simulink model "HvacMdlAirTDistbnDynFildFrnt"  (R2022b)
settings: Solver=FixedStepDiscrete  StartTime=0.0  StopTime=10.0  FixedStep=0.2  RelTol=1e-3
(part 1 of 2)
settings Model: Creator=blue.ge  LastModifiedBy=blue.ge
settings DataIOCC: SaveFormat=StructureWithTime
settings OptimizationCC: DefaultParameterBehavior=Inlined  EnhancedBackFolding=on  PassReuseOutputArgsAs=Individual arguments  ZeroExternalMemoryAtStartup=off  ZeroInternalMemoryAtStartup=off  OptimizeBlockOrder=speed  DifferentSizesBufferReuse=on  OptimizationCustomize=off  EfficientTunableParamExpr=on
settings DebuggingCC: ParameterTunabilityLossMsg=error
settings ModelReferenceCC: ModelReferenceNumInstancesAllowed=Single
settings SFSimCC: MATLABDynamicMemAlloc=off
settings RTWCC: Description=AUTOSAR  SystemTargetFile=autosar.tlc  GenCodeOnly=on  TemplateMakefile=ert_default_tmf
settings CodeAppCC: ForceParamTrailComments=on  IgnoreCustomStorageClasses=off  OperatorAnnotations=on  SimulinkDataObjDesc=on  SFDataObjDesc=on  InsertBlockDesc=on
settings STFCustomTargetCC: TargetLangStandard=C99 (ISO)  MultiwordLength=256  ParMdlRefBuildCompliant=on  CompOptLevelCompliant=on  IncludeMdlTerminateFcn=off  CombineOutputUpdateFcns=on  SuppressErrorStatus=on  ERTCustomFileBanners=on  SupportNonFinite=off  SupportComplex=off  SupportContinuousTime=off  SupportNonInlinedSFcns=off  RemoveResetFunc=on  MATLABClassNameForMDSCustomization=Simulink.SoftwareTarget.ERTCustomization  AutosarCompliant=on  UseToolchainInfoCompliant=on  LookupTableObjectStructAxisOrder=2,1,3,4,...  LUTObjectStructOrderEvenSpacing=Size,Table,Breakpoints  SystemTargetFile=autosar.tlc
settings ERTTargetCC: TargetLangStandard=C99 (ISO)  ConcurrentExecutionCompliant=off  IncludeMdlTerminateFcn=off  SuppressErrorStatus=on  SupportNonFinite=off  SupportComplex=off  ModelStepFunctionPrototypeControlCompliant=off  CPPClassGenCompliant=off  AutosarCompliant=on  LookupTableObjectStructAxisOrder=2,1,3,4,...  LUTObjectStructOrderEvenSpacing=Size,Table,Breakpoints  InlinedParameterPlacement=NonHierarchical  ERTCustomFileTemplate=example_file_process.tlc

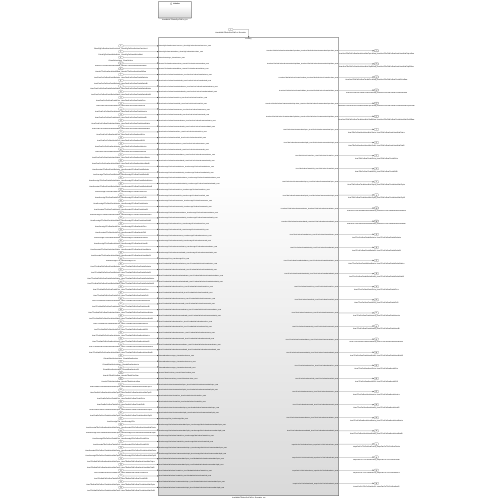
[thumbnail: Simulink's stored preview of the top-level diagram]
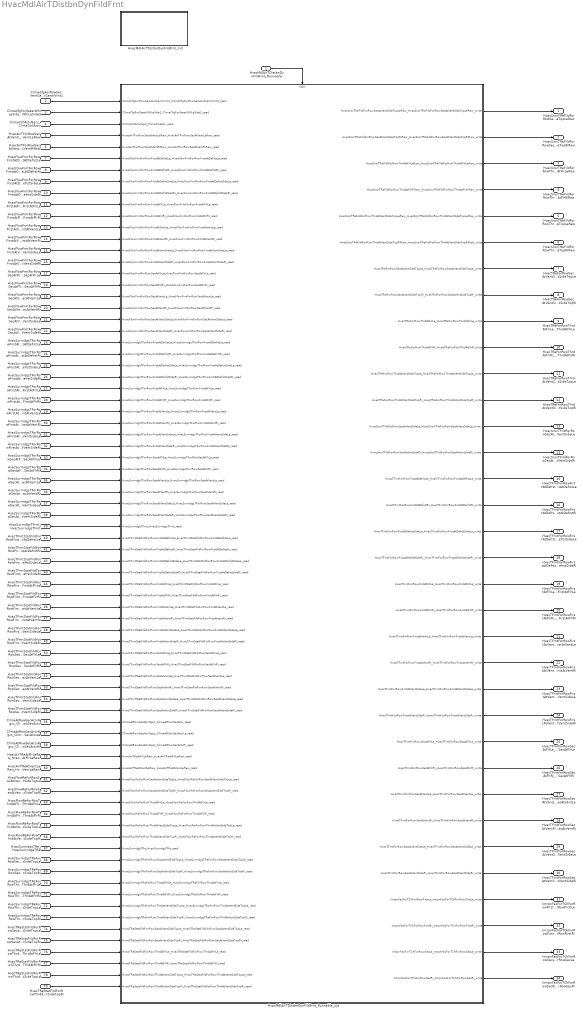
== system HvacMdlAirTDistbnDynFildFrnt ==
  HvacMdlAirTDistbnDynFildFrnt_Runnable : Inport  SampleTime=0.2  OutputFunctionCall=on
  ClimaCfgForRowSecVentCentrVrnt1_ClimaCfgForRowSecVentCentrVrnt1 : Inport  Port=2  OutDataTypeStr=Enum: ClimaCfgForRowSecVentCentrVrnt1  PortDimensions=1
  ClimaCfgForSteerWhlLeSide1_ClimaCfgForSteerWhlLeSide1 : Inport  Port=3  OutDataTypeStr=Boolean  PortDimensions=1
  ClimaCtrlActvNgcc_ClimaCtrlActv : Inport  Port=4  OutDataTypeStr=Boolean  PortDimensions=1
  HvacAirTForRowSecAtVentLeRaw_HvacAirTForRowSecAtVentLeRaw : Inport  Port=5  OutDataTypeStr=Bus: HvacAirTForRowSecAtVentLe  PortDimensions=1  BusOutputAsStruct=on
  HvacAirTForRowSecAtVentRiRaw_HvacAirTForRowSecAtVentRiRaw : Inport  Port=6  OutDataTypeStr=Bus: HvacAirTForRowSecAtVentRi  PortDimensions=1  BusOutputAsStruct=on
  HvacFlowFrntForRowFirstAtDefrstLe_HvacFlowFrntForRowFirstAtDefrstLe : Inport  Port=7  OutDataTypeStr=AirFlow30  PortDimensions=1
  HvacFlowFrntForRowFirstAtDefrstRi_HvacFlowFrntForRowFirstAtDefrstRi : Inport  Port=8  OutDataTypeStr=AirFlow30  PortDimensions=1
  HvacFlowFrntForRowFirstAtDefrstSideLe_HvacFlowFrntForRowFirstAtDefrstSideLe : Inport  Port=9  OutDataTypeStr=AirFlow30  PortDimensions=1
  HvacFlowFrntForRowFirstAtDefrstSideRi_HvacFlowFrntForRowFirstAtDefrstSideRi : Inport  Port=10  OutDataTypeStr=AirFlow30  PortDimensions=1
  HvacFlowFrntForRowFirstAtFlrLe_HvacFlowFrntForRowFirstAtFlrLe : Inport  Port=11  OutDataTypeStr=AirFlow30  PortDimensions=1
  HvacFlowFrntForRowFirstAtFlrRi_HvacFlowFrntForRowFirstAtFlrRi : Inport  Port=12  OutDataTypeStr=AirFlow30  PortDimensions=1
  HvacFlowFrntForRowFirstAtVentLe_HvacFlowFrntForRowFirstAtVentLe : Inport  Port=13  OutDataTypeStr=AirFlow30  PortDimensions=1
  HvacFlowFrntForRowFirstAtVentRi_HvacFlowFrntForRowFirstAtVentRi : Inport  Port=14  OutDataTypeStr=AirFlow30  PortDimensions=1
  HvacFlowFrntForRowFirstAtVentSideLe_HvacFlowFrntForRowFirstAtVentSideLe : Inport  Port=15  OutDataTypeStr=AirFlow30  PortDimensions=1
  HvacFlowFrntForRowFirstAtVentSideRi_HvacFlowFrntForRowFirstAtVentSideRi : Inport  Port=16  OutDataTypeStr=AirFlow30  PortDimensions=1
  HvacFlowFrntForRowSecAtFlrLe_HvacFlowFrntForRowSecAtFlrLe : Inport  Port=17  OutDataTypeStr=AirFlow30  PortDimensions=1
  HvacFlowFrntForRowSecAtFlrRi_HvacFlowFrntForRowSecAtFlrRi : Inport  Port=18  OutDataTypeStr=AirFlow30  PortDimensions=1
  HvacFlowFrntForRowSecAtVentLe_HvacFlowFrntForRowSecAtVentLe : Inport  Port=19  OutDataTypeStr=AirFlow30  PortDimensions=1
  HvacFlowFrntForRowSecAtVentRi_HvacFlowFrntForRowSecAtVentRi : Inport  Port=20  OutDataTypeStr=AirFlow30  PortDimensions=1
  HvacFlowFrntForRowSecAtVentSideLe_HvacFlowFrntForRowSecAtVentSideLe : Inport  Port=21  OutDataTypeStr=AirFlow30  PortDimensions=1
  HvacFlowFrntForRowSecAtVentSideRi_HvacFlowFrntForRowSecAtVentSideRi : Inport  Port=22  OutDataTypeStr=AirFlow30  PortDimensions=1
  HvacSurrndgsTForRowFirstAtDefrstLe_HvacSurrndgsTForRowFirstAtDefrstLe : Inport  Port=23  OutDataTypeStr=T30  PortDimensions=1
  HvacSurrndgsTForRowFirstAtDefrstRi_HvacSurrndgsTForRowFirstAtDefrstRi : Inport  Port=24  OutDataTypeStr=T30  PortDimensions=1
  HvacSurrndgsTForRowFirstAtDefrstSideLe_HvacSurrndgsTForRowFirstAtDefrstSideLe : Inport  Port=25  OutDataTypeStr=T30  PortDimensions=1
  HvacSurrndgsTForRowFirstAtDefrstSideRi_HvacSurrndgsTForRowFirstAtDefrstSideRi : Inport  Port=26  OutDataTypeStr=T30  PortDimensions=1
  HvacSurrndgsTForRowFirstAtFlrLe_HvacSurrndgsTForRowFirstAtFlrLe : Inport  Port=27  OutDataTypeStr=T30  PortDimensions=1
  HvacSurrndgsTForRowFirstAtFlrRi_HvacSurrndgsTForRowFirstAtFlrRi : Inport  Port=28  OutDataTypeStr=T30  PortDimensions=1
  HvacSurrndgsTForRowFirstAtVentLe_HvacSurrndgsTForRowFirstAtVentLe : Inport  Port=29  OutDataTypeStr=T30  PortDimensions=1
  HvacSurrndgsTForRowFirstAtVentRi_HvacSurrndgsTForRowFirstAtVentRi : Inport  Port=30  OutDataTypeStr=T30  PortDimensions=1
  HvacSurrndgsTForRowFirstAtVentSideLe_HvacSurrndgsTForRowFirstAtVentSideLe : Inport  Port=31  OutDataTypeStr=T30  PortDimensions=1
  HvacSurrndgsTForRowFirstAtVentSideRi_HvacSurrndgsTForRowFirstAtVentSideRi : Inport  Port=32  OutDataTypeStr=T30  PortDimensions=1
  HvacSurrndgsTForRowSecAtFlrLe_HvacSurrndgsTForRowSecAtFlrLe : Inport  Port=33  OutDataTypeStr=T30  PortDimensions=1
  HvacSurrndgsTForRowSecAtFlrRi_HvacSurrndgsTForRowSecAtFlrRi : Inport  Port=34  OutDataTypeStr=T30  PortDimensions=1
  HvacSurrndgsTForRowSecAtVentLe_HvacSurrndgsTForRowSecAtVentLe : Inport  Port=35  OutDataTypeStr=T30  PortDimensions=1
  HvacSurrndgsTForRowSecAtVentRi_HvacSurrndgsTForRowSecAtVentRi : Inport  Port=36  OutDataTypeStr=T30  PortDimensions=1
  HvacSurrndgsTForRowSecAtVentSideLe_HvacSurrndgsTForRowSecAtVentSideLe : Inport  Port=37  OutDataTypeStr=T30  PortDimensions=1
  HvacSurrndgsTForRowSecAtVentSideRi_HvacSurrndgsTForRowSecAtVentSideRi : Inport  Port=38  OutDataTypeStr=T30  PortDimensions=1
  HvacSurrndgsTFrnt_HvacSurrndgsTFrnt : Inport  Port=39  OutDataTypeStr=T30  PortDimensions=1
  HvacTFrntStatFildForRowFirstAtDefrstLe_HvacTFrntStatFildForRowFirstAtDefrstLe : Inport  Port=40  OutDataTypeStr=T30  PortDimensions=1
  HvacTFrntStatFildForRowFirstAtDefrstRi_HvacTFrntStatFildForRowFirstAtDefrstRi : Inport  Port=41  OutDataTypeStr=T30  PortDimensions=1
  HvacTFrntStatFildForRowFirstAtDefrstSideLe_HvacTFrntStatFildForRowFirstAtDefrstSideLe : Inport  Port=42  OutDataTypeStr=T30  PortDimensions=1
  HvacTFrntStatFildForRowFirstAtDefrstSideRi_HvacTFrntStatFildForRowFirstAtDefrstSideRi : Inport  Port=43  OutDataTypeStr=T30  PortDimensions=1
  HvacTFrntStatFildForRowFirstAtFlrLe_HvacTFrntStatFildForRowFirstAtFlrLe : Inport  Port=44  OutDataTypeStr=T30  PortDimensions=1
  HvacTFrntStatFildForRowFirstAtFlrRi_HvacTFrntStatFildForRowFirstAtFlrRi : Inport  Port=45  OutDataTypeStr=T30  PortDimensions=1
  HvacTFrntStatFildForRowFirstAtVentLe_HvacTFrntStatFildForRowFirstAtVentLe : Inport  Port=46  OutDataTypeStr=T30  PortDimensions=1
  HvacTFrntStatFildForRowFirstAtVentRi_HvacTFrntStatFildForRowFirstAtVentRi : Inport  Port=47  OutDataTypeStr=T30  PortDimensions=1
  HvacTFrntStatFildForRowFirstAtVentSideLe_HvacTFrntStatFildForRowFirstAtVentSideLe : Inport  Port=48  OutDataTypeStr=T30  PortDimensions=1
  HvacTFrntStatFildForRowFirstAtVentSideRi_HvacTFrntStatFildForRowFirstAtVentSideRi : Inport  Port=49  OutDataTypeStr=T30  PortDimensions=1
  HvacTFrntStatFildForRowSecAtFlrLe_HvacTFrntStatFildForRowSecAtFlrLe : Inport  Port=50  OutDataTypeStr=T30  PortDimensions=1
  HvacTFrntStatFildForRowSecAtFlrRi_HvacTFrntStatFildForRowSecAtFlrRi : Inport  Port=51  OutDataTypeStr=T30  PortDimensions=1
  HvacTFrntStatFildForRowSecAtVentLe_HvacTFrntStatFildForRowSecAtVentLe : Inport  Port=52  OutDataTypeStr=T30  PortDimensions=1
  HvacTFrntStatFildForRowSecAtVentRi_HvacTFrntStatFildForRowSecAtVentRi : Inport  Port=53  OutDataTypeStr=T30  PortDimensions=1
  HvacTFrntStatFildForRowSecAtVentSideLe_HvacTFrntStatFildForRowSecAtVentSideLe : Inport  Port=54  OutDataTypeStr=T30  PortDimensions=1
  HvacTFrntStatFildForRowSecAtVentSideRi_HvacTFrntStatFildForRowSecAtVentSideRi : Inport  Port=55  OutDataTypeStr=T30  PortDimensions=1
  ClimaAtRowSecActvNgcc_ClimaAtRowSecActv : Inport  Port=56  OutDataTypeStr=Boolean  PortDimensions=1
  ClimaAtRowSecActvNgcc_ClimaAtRowSecActvLe : Inport  Port=57  OutDataTypeStr=Boolean  PortDimensions=1
  ClimaAtRowSecActvNgcc_ClimaAtRowSecActvRi : Inport  Port=58  OutDataTypeStr=Boolean  PortDimensions=1
  HvacAirTReAtFlrLeRaw_HvacAirTReAtFlrLeRaw : Inport  Port=59  OutDataTypeStr=Bus: HvacAirTReAtFlrLe  PortDimensions=1  BusOutputAsStruct=on
  HvacAirTReAtVentLeRaw_HvacAirTReAtVentLeRaw : Inport  Port=60  OutDataTypeStr=Bus: HvacAirTReAtVentLe  PortDimensions=1  BusOutputAsStruct=on
  HvacFlowReForRowSecAtVentSideTopLe_HvacFlowReForRowSecAtVentSideTopLe : Inport  Port=61  OutDataTypeStr=AirFlow30  PortDimensions=1
  HvacFlowReForRowSecAtVentSideTopRi_HvacFlowReForRowSecAtVentSideTopRi : Inport  Port=62  OutDataTypeStr=AirFlow30  PortDimensions=1
  HvacFlowReForRowThrdAtFlrLe_HvacFlowReForRowThrdAtFlrLe : Inport  Port=63  OutDataTypeStr=AirFlow30  PortDimensions=1
  HvacFlowReForRowThrdAtFlrRi_HvacFlowReForRowThrdAtFlrRi : Inport  Port=64  OutDataTypeStr=AirFlow30  PortDimensions=1
  HvacFlowReForRowThrdAtVentSideTopLe_HvacFlowReForRowThrdAtVentSideTopLe : Inport  Port=65  OutDataTypeStr=AirFlow30  PortDimensions=1
  HvacFlowReForRowThrdAtVentSideTopRi_HvacFlowReForRowThrdAtVentSideTopRi : Inport  Port=66  OutDataTypeStr=AirFlow30  PortDimensions=1
  HvacSurrndgsTRe_HvacSurrndgsTRe : Inport  Port=67  OutDataTypeStr=T30  PortDimensions=1
  HvacSurrndgsTReForRowSecAtVentSideTopLe_HvacSurrndgsTReForRowSecAtVentSideTopLe : Inport  Port=68  OutDataTypeStr=T30  PortDimensions=1
  HvacSurrndgsTReForRowSecAtVentSideTopRi_HvacSurrndgsTReForRowSecAtVentSideTopRi : Inport  Port=69  OutDataTypeStr=T30  PortDimensions=1
  HvacSurrndgsTReForRowThrdAtFlrLe_HvacSurrndgsTReForRowThrdAtFlrLe : Inport  Port=70  OutDataTypeStr=T30  PortDimensions=1
  HvacSurrndgsTReForRowThrdAtFlrRi_HvacSurrndgsTReForRowThrdAtFlrRi : Inport  Port=71  OutDataTypeStr=T30  PortDimensions=1
  HvacSurrndgsTReForRowThrdAtVentSideTopLe_HvacSurrndgsTReForRowThrdAtVentSideTopLe : Inport  Port=72  OutDataTypeStr=T30  PortDimensions=1
  HvacSurrndgsTReForRowThrdAtVentSideTopRi_HvacSurrndgsTReForRowThrdAtVentSideTopRi : Inport  Port=73  OutDataTypeStr=T30  PortDimensions=1
  HvacTReStatFildForRowSecAtVentSideTopLe_HvacTReStatFildForRowSecAtVentSideTopLe : Inport  Port=74  OutDataTypeStr=T30  PortDimensions=1
  HvacTReStatFildForRowSecAtVentSideTopRi_HvacTReStatFildForRowSecAtVentSideTopRi : Inport  Port=75  OutDataTypeStr=T30  PortDimensions=1
  HvacTReStatFildForRowThrdAtFlrLe_HvacTReStatFildForRowThrdAtFlrLe : Inport  Port=76  OutDataTypeStr=T30  PortDimensions=1
  HvacTReStatFildForRowThrdAtFlrRi_HvacTReStatFildForRowThrdAtFlrRi : Inport  Port=77  OutDataTypeStr=T30  PortDimensions=1
  HvacTReStatFildForRowThrdAtVentSideTopLe_HvacTReStatFildForRowThrdAtVentSideTopLe : Inport  Port=78  OutDataTypeStr=T30  PortDimensions=1
  HvacTReStatFildForRowThrdAtVentSideTopRi_HvacTReStatFildForRowThrdAtVentSideTopRi : Inport  Port=79  OutDataTypeStr=T30  PortDimensions=1
  HvacMdlAirTDistbnDynFildFrnt_Init : Subsystem
  HvacMdlAirTDistbnDynFildFrnt_Runnable_sys : Subsystem
  HvacSnsrTReFildForRowSecAtVentSideTopLeRaw_HvacSnsrTReFildForRowSecAtVentSideTopLeRaw : Outport  OutDataTypeStr=Bus: HvacAirTReForRowSecAtVentSideTopLe  PortDimensions=1  BusOutputAsStruct=on
  HvacSnsrTReFildForRowSecAtVentSideTopRiRaw_HvacSnsrTReFildForRowSecAtVentSideTopRiRaw : Outport  Port=2  OutDataTypeStr=Bus: HvacAirTReForRowSecAtVentSideTopRi  PortDimensions=1  BusOutputAsStruct=on
  HvacSnsrTReFildForRowThrdAtFlrLeRaw_HvacSnsrTReFildForRowThrdAtFlrLeRaw : Outport  Port=3  OutDataTypeStr=Bus: HvacAirTReForRowThrdAtFlrLe  PortDimensions=1  BusOutputAsStruct=on
  HvacSnsrTReFildForRowThrdAtFlrRiRaw_HvacSnsrTReFildForRowThrdAtFlrRiRaw : Outport  Port=4  OutDataTypeStr=Bus: HvacAirTReForRowThrdAtFlrRi  PortDimensions=1  BusOutputAsStruct=on
  HvacSnsrTReFildForRowThrdAtVentSideTopLeRaw_HvacSnsrTReFildForRowThrdAtVentSideTopLeRaw : Outport  Port=5  OutDataTypeStr=Bus: HvacAirTReForRowThrdAtVentSideTopLe  PortDimensions=1  BusOutputAsStruct=on
  HvacSnsrTReFildForRowThrdAtVentSideTopRiRaw_HvacSnsrTReFildForRowThrdAtVentSideTopRiRaw : Outport  Port=6  OutDataTypeStr=Bus: HvacAirTReForRowThrdAtVentSideTopRi  PortDimensions=1  BusOutputAsStruct=on
  HvacTReForRowSecAtVentSideTopLe_HvacTReForRowSecAtVentSideTopLe : Outport  Port=7  OutDataTypeStr=T30  PortDimensions=1
  HvacTReForRowSecAtVentSideTopRi_HvacTReForRowSecAtVentSideTopRi : Outport  Port=8  OutDataTypeStr=T30  PortDimensions=1
  HvacTReForRowThrdAtFlrLe_HvacTReForRowThrdAtFlrLe : Outport  Port=9  OutDataTypeStr=T30  PortDimensions=1
  HvacTReForRowThrdAtFlrRi_HvacTReForRowThrdAtFlrRi : Outport  Port=10  OutDataTypeStr=T30  PortDimensions=1
  HvacTReForRowThrdAtVentSideTopLe_HvacTReForRowThrdAtVentSideTopLe : Outport  Port=11  OutDataTypeStr=T30  PortDimensions=1
  HvacTReForRowThrdAtVentSideTopRi_HvacTReForRowThrdAtVentSideTopRi : Outport  Port=12  OutDataTypeStr=T30  PortDimensions=1
  HvacSnsrTFildForRowSecAtVentSideLe_HvacSnsrTFildForRowSecAtVentSideLe : Outport  Port=13  OutDataTypeStr=Bus: HvacAirTForRowSecAtVentLe  PortDimensions=1  BusOutputAsStruct=on
  HvacSnsrTFildForRowSecAtVentSideRi_HvacSnsrTFildForRowSecAtVentSideRi : Outport  Port=14  OutDataTypeStr=Bus: HvacAirTForRowSecAtVentRi  PortDimensions=1  BusOutputAsStruct=on
  HvacTFrntForRowFirstAtDefrstLe_HvacTFrntForRowFirstAtDefrstLe : Outport  Port=15  OutDataTypeStr=T30  PortDimensions=1
  HvacTFrntForRowFirstAtDefrstRi_HvacTFrntForRowFirstAtDefrstRi : Outport  Port=16  OutDataTypeStr=T30  PortDimensions=1
  HvacTFrntForRowFirstAtDefrstSideLe_HvacTFrntForRowFirstAtDefrstSideLe : Outport  Port=17  OutDataTypeStr=T30  PortDimensions=1
  HvacTFrntForRowFirstAtDefrstSideRi_HvacTFrntForRowFirstAtDefrstSideRi : Outport  Port=18  OutDataTypeStr=T30  PortDimensions=1
  HvacTFrntForRowFirstAtFlrLe_HvacTFrntForRowFirstAtFlrLe : Outport  Port=19  OutDataTypeStr=T30  PortDimensions=1
  HvacTFrntForRowFirstAtFlrRi_HvacTFrntForRowFirstAtFlrRi : Outport  Port=20  OutDataTypeStr=T30  PortDimensions=1
  HvacTFrntForRowFirstAtVentLe_HvacTFrntForRowFirstAtVentLe : Outport  Port=21  OutDataTypeStr=T30  PortDimensions=1
  HvacTFrntForRowFirstAtVentRi_HvacTFrntForRowFirstAtVentRi : Outport  Port=22  OutDataTypeStr=T30  PortDimensions=1
  HvacTFrntForRowFirstAtVentSideLe_HvacTFrntForRowFirstAtVentSideLe : Outport  Port=23  OutDataTypeStr=T30  PortDimensions=1
  HvacTFrntForRowFirstAtVentSideRi_HvacTFrntForRowFirstAtVentSideRi : Outport  Port=24  OutDataTypeStr=T30  PortDimensions=1
  HvacTFrntForRowSecAtFlrLe_HvacTFrntForRowSecAtFlrLe : Outport  Port=25  OutDataTypeStr=T30  PortDimensions=1
  HvacTFrntForRowSecAtFlrRi_HvacTFrntForRowSecAtFlrRi : Outport  Port=26  OutDataTypeStr=T30  PortDimensions=1
  HvacTFrntForRowSecAtVentLe_HvacTFrntForRowSecAtVentLe : Outport  Port=27  OutDataTypeStr=T30  PortDimensions=1
  HvacTFrntForRowSecAtVentRi_HvacTFrntForRowSecAtVentRi : Outport  Port=28  OutDataTypeStr=T30  PortDimensions=1
  HvacTFrntForRowSecAtVentSideLe_HvacTFrntForRowSecAtVentSideLe : Outport  Port=29  OutDataTypeStr=T30  PortDimensions=1
  HvacTFrntForRowSecAtVentSideRi_HvacTFrntForRowSecAtVentSideRi : Outport  Port=30  OutDataTypeStr=T30  PortDimensions=1
  IntrpnFacForTChForRowFirstLe_IntrpnFacForTChForRowFirstLe : Outport  Port=31  OutDataTypeStr=Fac30  PortDimensions=1
  IntrpnFacForTChForRowFirstRi_IntrpnFacForTChForRowFirstRi : Outport  Port=32  OutDataTypeStr=Fac30  PortDimensions=1
  IntrpnFacForTChForRowSecLe_IntrpnFacForTChForRowSecLe : Outport  Port=33  OutDataTypeStr=Fac30  PortDimensions=1
  IntrpnFacForTChForRowSecRi_IntrpnFacForTChForRowSecRi : Outport  Port=34  OutDataTypeStr=Fac30  PortDimensions=1
  wires:
  ClimaCfgForRowSecVentCentrVrnt1_ClimaCfgForRowSecVentCentrVrnt1/1 -> HvacMdlAirTDistbnDynFildFrnt_Runnable_sys/1(ClimaCfgForRowSecVentCentrVrnt1_ClimaCfgForRowSecVentCentrVrnt1_read)
  ClimaCfgForSteerWhlLeSide1_ClimaCfgForSteerWhlLeSide1/1 -> HvacMdlAirTDistbnDynFildFrnt_Runnable_sys/2(ClimaCfgForSteerWhlLeSide1_ClimaCfgForSteerWhlLeSide1_read)
  ClimaCtrlActvNgcc_ClimaCtrlActv/1 -> HvacMdlAirTDistbnDynFildFrnt_Runnable_sys/3(ClimaCtrlActvNgcc_ClimaCtrlActv_read)
  HvacAirTForRowSecAtVentLeRaw_HvacAirTForRowSecAtVentLeRaw/1 -> HvacMdlAirTDistbnDynFildFrnt_Runnable_sys/4(HvacAirTForRowSecAtVentLeRaw_HvacAirTForRowSecAtVentLeRaw_read)
  HvacAirTForRowSecAtVentRiRaw_HvacAirTForRowSecAtVentRiRaw/1 -> HvacMdlAirTDistbnDynFildFrnt_Runnable_sys/5(HvacAirTForRowSecAtVentRiRaw_HvacAirTForRowSecAtVentRiRaw_read)
  HvacFlowFrntForRowFirstAtDefrstLe_HvacFlowFrntForRowFirstAtDefrstLe/1 -> HvacMdlAirTDistbnDynFildFrnt_Runnable_sys/6(HvacFlowFrntForRowFirstAtDefrstLe_HvacFlowFrntForRowFirstAtDefrstLe_read)
  HvacFlowFrntForRowFirstAtDefrstRi_HvacFlowFrntForRowFirstAtDefrstRi/1 -> HvacMdlAirTDistbnDynFildFrnt_Runnable_sys/7(HvacFlowFrntForRowFirstAtDefrstRi_HvacFlowFrntForRowFirstAtDefrstRi_read)
  HvacFlowFrntForRowFirstAtDefrstSideLe_HvacFlowFrntForRowFirstAtDefrstSideLe/1 -> HvacMdlAirTDistbnDynFildFrnt_Runnable_sys/8(HvacFlowFrntForRowFirstAtDefrstSideLe_HvacFlowFrntForRowFirstAtDefrstSideLe_read)
  HvacFlowFrntForRowFirstAtDefrstSideRi_HvacFlowFrntForRowFirstAtDefrstSideRi/1 -> HvacMdlAirTDistbnDynFildFrnt_Runnable_sys/9(HvacFlowFrntForRowFirstAtDefrstSideRi_HvacFlowFrntForRowFirstAtDefrstSideRi_read)
  HvacFlowFrntForRowFirstAtFlrLe_HvacFlowFrntForRowFirstAtFlrLe/1 -> HvacMdlAirTDistbnDynFildFrnt_Runnable_sys/10(HvacFlowFrntForRowFirstAtFlrLe_HvacFlowFrntForRowFirstAtFlrLe_read)
  HvacFlowFrntForRowFirstAtFlrRi_HvacFlowFrntForRowFirstAtFlrRi/1 -> HvacMdlAirTDistbnDynFildFrnt_Runnable_sys/11(HvacFlowFrntForRowFirstAtFlrRi_HvacFlowFrntForRowFirstAtFlrRi_read)
  HvacFlowFrntForRowFirstAtVentLe_HvacFlowFrntForRowFirstAtVentLe/1 -> HvacMdlAirTDistbnDynFildFrnt_Runnable_sys/12(HvacFlowFrntForRowFirstAtVentLe_HvacFlowFrntForRowFirstAtVentLe_read)
  HvacFlowFrntForRowFirstAtVentRi_HvacFlowFrntForRowFirstAtVentRi/1 -> HvacMdlAirTDistbnDynFildFrnt_Runnable_sys/13(HvacFlowFrntForRowFirstAtVentRi_HvacFlowFrntForRowFirstAtVentRi_read)
  HvacFlowFrntForRowFirstAtVentSideLe_HvacFlowFrntForRowFirstAtVentSideLe/1 -> HvacMdlAirTDistbnDynFildFrnt_Runnable_sys/14(HvacFlowFrntForRowFirstAtVentSideLe_HvacFlowFrntForRowFirstAtVentSideLe_read)
  HvacFlowFrntForRowFirstAtVentSideRi_HvacFlowFrntForRowFirstAtVentSideRi/1 -> HvacMdlAirTDistbnDynFildFrnt_Runnable_sys/15(HvacFlowFrntForRowFirstAtVentSideRi_HvacFlowFrntForRowFirstAtVentSideRi_read)
  HvacFlowFrntForRowSecAtFlrLe_HvacFlowFrntForRowSecAtFlrLe/1 -> HvacMdlAirTDistbnDynFildFrnt_Runnable_sys/16(HvacFlowFrntForRowSecAtFlrLe_HvacFlowFrntForRowSecAtFlrLe_read)
  HvacFlowFrntForRowSecAtFlrRi_HvacFlowFrntForRowSecAtFlrRi/1 -> HvacMdlAirTDistbnDynFildFrnt_Runnable_sys/17(HvacFlowFrntForRowSecAtFlrRi_HvacFlowFrntForRowSecAtFlrRi_read)
  HvacFlowFrntForRowSecAtVentLe_HvacFlowFrntForRowSecAtVentLe/1 -> HvacMdlAirTDistbnDynFildFrnt_Runnable_sys/18(HvacFlowFrntForRowSecAtVentLe_HvacFlowFrntForRowSecAtVentLe_read)
  HvacFlowFrntForRowSecAtVentRi_HvacFlowFrntForRowSecAtVentRi/1 -> HvacMdlAirTDistbnDynFildFrnt_Runnable_sys/19(HvacFlowFrntForRowSecAtVentRi_HvacFlowFrntForRowSecAtVentRi_read)
  HvacFlowFrntForRowSecAtVentSideLe_HvacFlowFrntForRowSecAtVentSideLe/1 -> HvacMdlAirTDistbnDynFildFrnt_Runnable_sys/20(HvacFlowFrntForRowSecAtVentSideLe_HvacFlowFrntForRowSecAtVentSideLe_read)
  HvacFlowFrntForRowSecAtVentSideRi_HvacFlowFrntForRowSecAtVentSideRi/1 -> HvacMdlAirTDistbnDynFildFrnt_Runnable_sys/21(HvacFlowFrntForRowSecAtVentSideRi_HvacFlowFrntForRowSecAtVentSideRi_read)
  HvacSurrndgsTForRowFirstAtDefrstLe_HvacSurrndgsTForRowFirstAtDefrstLe/1 -> HvacMdlAirTDistbnDynFildFrnt_Runnable_sys/22(HvacSurrndgsTForRowFirstAtDefrstLe_HvacSurrndgsTForRowFirstAtDefrstLe_read)
  HvacSurrndgsTForRowFirstAtDefrstRi_HvacSurrndgsTForRowFirstAtDefrstRi/1 -> HvacMdlAirTDistbnDynFildFrnt_Runnable_sys/23(HvacSurrndgsTForRowFirstAtDefrstRi_HvacSurrndgsTForRowFirstAtDefrstRi_read)
  HvacSurrndgsTForRowFirstAtDefrstSideLe_HvacSurrndgsTForRowFirstAtDefrstSideLe/1 -> HvacMdlAirTDistbnDynFildFrnt_Runnable_sys/24(HvacSurrndgsTForRowFirstAtDefrstSideLe_HvacSurrndgsTForRowFirstAtDefrstSideLe_read)
  HvacSurrndgsTForRowFirstAtDefrstSideRi_HvacSurrndgsTForRowFirstAtDefrstSideRi/1 -> HvacMdlAirTDistbnDynFildFrnt_Runnable_sys/25(HvacSurrndgsTForRowFirstAtDefrstSideRi_HvacSurrndgsTForRowFirstAtDefrstSideRi_read)
  HvacSurrndgsTForRowFirstAtFlrLe_HvacSurrndgsTForRowFirstAtFlrLe/1 -> HvacMdlAirTDistbnDynFildFrnt_Runnable_sys/26(HvacSurrndgsTForRowFirstAtFlrLe_HvacSurrndgsTForRowFirstAtFlrLe_read)
  HvacSurrndgsTForRowFirstAtFlrRi_HvacSurrndgsTForRowFirstAtFlrRi/1 -> HvacMdlAirTDistbnDynFildFrnt_Runnable_sys/27(HvacSurrndgsTForRowFirstAtFlrRi_HvacSurrndgsTForRowFirstAtFlrRi_read)
  HvacSurrndgsTForRowFirstAtVentLe_HvacSurrndgsTForRowFirstAtVentLe/1 -> HvacMdlAirTDistbnDynFildFrnt_Runnable_sys/28(HvacSurrndgsTForRowFirstAtVentLe_HvacSurrndgsTForRowFirstAtVentLe_read)
  HvacSurrndgsTForRowFirstAtVentRi_HvacSurrndgsTForRowFirstAtVentRi/1 -> HvacMdlAirTDistbnDynFildFrnt_Runnable_sys/29(HvacSurrndgsTForRowFirstAtVentRi_HvacSurrndgsTForRowFirstAtVentRi_read)
  HvacSurrndgsTForRowFirstAtVentSideLe_HvacSurrndgsTForRowFirstAtVentSideLe/1 -> HvacMdlAirTDistbnDynFildFrnt_Runnable_sys/30(HvacSurrndgsTForRowFirstAtVentSideLe_HvacSurrndgsTForRowFirstAtVentSideLe_read)
  HvacSurrndgsTForRowFirstAtVentSideRi_HvacSurrndgsTForRowFirstAtVentSideRi/1 -> HvacMdlAirTDistbnDynFildFrnt_Runnable_sys/31(HvacSurrndgsTForRowFirstAtVentSideRi_HvacSurrndgsTForRowFirstAtVentSideRi_read)
  HvacSurrndgsTForRowSecAtFlrLe_HvacSurrndgsTForRowSecAtFlrLe/1 -> HvacMdlAirTDistbnDynFildFrnt_Runnable_sys/32(HvacSurrndgsTForRowSecAtFlrLe_HvacSurrndgsTForRowSecAtFlrLe_read)
  HvacSurrndgsTForRowSecAtFlrRi_HvacSurrndgsTForRowSecAtFlrRi/1 -> HvacMdlAirTDistbnDynFildFrnt_Runnable_sys/33(HvacSurrndgsTForRowSecAtFlrRi_HvacSurrndgsTForRowSecAtFlrRi_read)
  HvacSurrndgsTForRowSecAtVentLe_HvacSurrndgsTForRowSecAtVentLe/1 -> HvacMdlAirTDistbnDynFildFrnt_Runnable_sys/34(HvacSurrndgsTForRowSecAtVentLe_HvacSurrndgsTForRowSecAtVentLe_read)
  HvacSurrndgsTForRowSecAtVentRi_HvacSurrndgsTForRowSecAtVentRi/1 -> HvacMdlAirTDistbnDynFildFrnt_Runnable_sys/35(HvacSurrndgsTForRowSecAtVentRi_HvacSurrndgsTForRowSecAtVentRi_read)
  HvacSurrndgsTForRowSecAtVentSideLe_HvacSurrndgsTForRowSecAtVentSideLe/1 -> HvacMdlAirTDistbnDynFildFrnt_Runnable_sys/36(HvacSurrndgsTForRowSecAtVentSideLe_HvacSurrndgsTForRowSecAtVentSideLe_read)
  HvacSurrndgsTForRowSecAtVentSideRi_HvacSurrndgsTForRowSecAtVentSideRi/1 -> HvacMdlAirTDistbnDynFildFrnt_Runnable_sys/37(HvacSurrndgsTForRowSecAtVentSideRi_HvacSurrndgsTForRowSecAtVentSideRi_read)
  HvacSurrndgsTFrnt_HvacSurrndgsTFrnt/1 -> HvacMdlAirTDistbnDynFildFrnt_Runnable_sys/38(HvacSurrndgsTFrnt_HvacSurrndgsTFrnt_read)
  HvacTFrntStatFildForRowFirstAtDefrstLe_HvacTFrntStatFildForRowFirstAtDefrstLe/1 -> HvacMdlAirTDistbnDynFildFrnt_Runnable_sys/39(HvacTFrntStatFildForRowFirstAtDefrstLe_HvacTFrntStatFildForRowFirstAtDefrstLe_read)
  HvacTFrntStatFildForRowFirstAtDefrstRi_HvacTFrntStatFildForRowFirstAtDefrstRi/1 -> HvacMdlAirTDistbnDynFildFrnt_Runnable_sys/40(HvacTFrntStatFildForRowFirstAtDefrstRi_HvacTFrntStatFildForRowFirstAtDefrstRi_read)
  HvacTFrntStatFildForRowFirstAtDefrstSideLe_HvacTFrntStatFildForRowFirstAtDefrstSideLe/1 -> HvacMdlAirTDistbnDynFildFrnt_Runnable_sys/41(HvacTFrntStatFildForRowFirstAtDefrstSideLe_HvacTFrntStatFildForRowFirstAtDefrstSideLe_read)
  HvacTFrntStatFildForRowFirstAtDefrstSideRi_HvacTFrntStatFildForRowFirstAtDefrstSideRi/1 -> HvacMdlAirTDistbnDynFildFrnt_Runnable_sys/42(HvacTFrntStatFildForRowFirstAtDefrstSideRi_HvacTFrntStatFildForRowFirstAtDefrstSideRi_read)
  HvacTFrntStatFildForRowFirstAtFlrLe_HvacTFrntStatFildForRowFirstAtFlrLe/1 -> HvacMdlAirTDistbnDynFildFrnt_Runnable_sys/43(HvacTFrntStatFildForRowFirstAtFlrLe_HvacTFrntStatFildForRowFirstAtFlrLe_read)
  HvacTFrntStatFildForRowFirstAtFlrRi_HvacTFrntStatFildForRowFirstAtFlrRi/1 -> HvacMdlAirTDistbnDynFildFrnt_Runnable_sys/44(HvacTFrntStatFildForRowFirstAtFlrRi_HvacTFrntStatFildForRowFirstAtFlrRi_read)
  HvacTFrntStatFildForRowFirstAtVentLe_HvacTFrntStatFildForRowFirstAtVentLe/1 -> HvacMdlAirTDistbnDynFildFrnt_Runnable_sys/45(HvacTFrntStatFildForRowFirstAtVentLe_HvacTFrntStatFildForRowFirstAtVentLe_read)
  HvacTFrntStatFildForRowFirstAtVentRi_HvacTFrntStatFildForRowFirstAtVentRi/1 -> HvacMdlAirTDistbnDynFildFrnt_Runnable_sys/46(HvacTFrntStatFildForRowFirstAtVentRi_HvacTFrntStatFildForRowFirstAtVentRi_read)
  HvacTFrntStatFildForRowFirstAtVentSideLe_HvacTFrntStatFildForRowFirstAtVentSideLe/1 -> HvacMdlAirTDistbnDynFildFrnt_Runnable_sys/47(HvacTFrntStatFildForRowFirstAtVentSideLe_HvacTFrntStatFildForRowFirstAtVentSideLe_read)
  HvacTFrntStatFildForRowFirstAtVentSideRi_HvacTFrntStatFildForRowFirstAtVentSideRi/1 -> HvacMdlAirTDistbnDynFildFrnt_Runnable_sys/48(HvacTFrntStatFildForRowFirstAtVentSideRi_HvacTFrntStatFildForRowFirstAtVentSideRi_read)
  HvacTFrntStatFildForRowSecAtFlrLe_HvacTFrntStatFildForRowSecAtFlrLe/1 -> HvacMdlAirTDistbnDynFildFrnt_Runnable_sys/49(HvacTFrntStatFildForRowSecAtFlrLe_HvacTFrntStatFildForRowSecAtFlrLe_read)
  HvacTFrntStatFildForRowSecAtFlrRi_HvacTFrntStatFildForRowSecAtFlrRi/1 -> HvacMdlAirTDistbnDynFildFrnt_Runnable_sys/50(HvacTFrntStatFildForRowSecAtFlrRi_HvacTFrntStatFildForRowSecAtFlrRi_read)
  HvacTFrntStatFildForRowSecAtVentLe_HvacTFrntStatFildForRowSecAtVentLe/1 -> HvacMdlAirTDistbnDynFildFrnt_Runnable_sys/51(HvacTFrntStatFildForRowSecAtVentLe_HvacTFrntStatFildForRowSecAtVentLe_read)
  HvacTFrntStatFildForRowSecAtVentRi_HvacTFrntStatFildForRowSecAtVentRi/1 -> HvacMdlAirTDistbnDynFildFrnt_Runnable_sys/52(HvacTFrntStatFildForRowSecAtVentRi_HvacTFrntStatFildForRowSecAtVentRi_read)
  HvacTFrntStatFildForRowSecAtVentSideLe_HvacTFrntStatFildForRowSecAtVentSideLe/1 -> HvacMdlAirTDistbnDynFildFrnt_Runnable_sys/53(HvacTFrntStatFildForRowSecAtVentSideLe_HvacTFrntStatFildForRowSecAtVentSideLe_read)
  HvacTFrntStatFildForRowSecAtVentSideRi_HvacTFrntStatFildForRowSecAtVentSideRi/1 -> HvacMdlAirTDistbnDynFildFrnt_Runnable_sys/54(HvacTFrntStatFildForRowSecAtVentSideRi_HvacTFrntStatFildForRowSecAtVentSideRi_read)
  ClimaAtRowSecActvNgcc_ClimaAtRowSecActv/1 -> HvacMdlAirTDistbnDynFildFrnt_Runnable_sys/55(ClimaAtRowSecActvNgcc_ClimaAtRowSecActv_read)
  ClimaAtRowSecActvNgcc_ClimaAtRowSecActvLe/1 -> HvacMdlAirTDistbnDynFildFrnt_Runnable_sys/56(ClimaAtRowSecActvNgcc_ClimaAtRowSecActvLe_read)
  ClimaAtRowSecActvNgcc_ClimaAtRowSecActvRi/1 -> HvacMdlAirTDistbnDynFildFrnt_Runnable_sys/57(ClimaAtRowSecActvNgcc_ClimaAtRowSecActvRi_read)
  HvacAirTReAtFlrLeRaw_HvacAirTReAtFlrLeRaw/1 -> HvacMdlAirTDistbnDynFildFrnt_Runnable_sys/58(HvacAirTReAtFlrLeRaw_HvacAirTReAtFlrLeRaw_read)
  HvacAirTReAtVentLeRaw_HvacAirTReAtVentLeRaw/1 -> HvacMdlAirTDistbnDynFildFrnt_Runnable_sys/59(HvacAirTReAtVentLeRaw_HvacAirTReAtVentLeRaw_read)
  HvacFlowReForRowSecAtVentSideTopLe_HvacFlowReForRowSecAtVentSideTopLe/1 -> HvacMdlAirTDistbnDynFildFrnt_Runnable_sys/60(HvacFlowReForRowSecAtVentSideTopLe_HvacFlowReForRowSecAtVentSideTopLe_read)
  HvacFlowReForRowSecAtVentSideTopRi_HvacFlowReForRowSecAtVentSideTopRi/1 -> HvacMdlAirTDistbnDynFildFrnt_Runnable_sys/61(HvacFlowReForRowSecAtVentSideTopRi_HvacFlowReForRowSecAtVentSideTopRi_read)
  HvacFlowReForRowThrdAtFlrLe_HvacFlowReForRowThrdAtFlrLe/1 -> HvacMdlAirTDistbnDynFildFrnt_Runnable_sys/62(HvacFlowReForRowThrdAtFlrLe_HvacFlowReForRowThrdAtFlrLe_read)
  HvacFlowReForRowThrdAtFlrRi_HvacFlowReForRowThrdAtFlrRi/1 -> HvacMdlAirTDistbnDynFildFrnt_Runnable_sys/63(HvacFlowReForRowThrdAtFlrRi_HvacFlowReForRowThrdAtFlrRi_read)
  HvacFlowReForRowThrdAtVentSideTopLe_HvacFlowReForRowThrdAtVentSideTopLe/1 -> HvacMdlAirTDistbnDynFildFrnt_Runnable_sys/64(HvacFlowReForRowThrdAtVentSideTopLe_HvacFlowReForRowThrdAtVentSideTopLe_read)
  HvacFlowReForRowThrdAtVentSideTopRi_HvacFlowReForRowThrdAtVentSideTopRi/1 -> HvacMdlAirTDistbnDynFildFrnt_Runnable_sys/65(HvacFlowReForRowThrdAtVentSideTopRi_HvacFlowReForRowThrdAtVentSideTopRi_read)
  HvacSurrndgsTRe_HvacSurrndgsTRe/1 -> HvacMdlAirTDistbnDynFildFrnt_Runnable_sys/66(HvacSurrndgsTRe_HvacSurrndgsTRe_read)
  HvacSurrndgsTReForRowSecAtVentSideTopLe_HvacSurrndgsTReForRowSecAtVentSideTopLe/1 -> HvacMdlAirTDistbnDynFildFrnt_Runnable_sys/67(HvacSurrndgsTReForRowSecAtVentSideTopLe_HvacSurrndgsTReForRowSecAtVentSideTopLe_read)
  HvacSurrndgsTReForRowSecAtVentSideTopRi_HvacSurrndgsTReForRowSecAtVentSideTopRi/1 -> HvacMdlAirTDistbnDynFildFrnt_Runnable_sys/68(HvacSurrndgsTReForRowSecAtVentSideTopRi_HvacSurrndgsTReForRowSecAtVentSideTopRi_read)
  HvacSurrndgsTReForRowThrdAtFlrLe_HvacSurrndgsTReForRowThrdAtFlrLe/1 -> HvacMdlAirTDistbnDynFildFrnt_Runnable_sys/69(HvacSurrndgsTReForRowThrdAtFlrLe_HvacSurrndgsTReForRowThrdAtFlrLe_read)
  HvacSurrndgsTReForRowThrdAtFlrRi_HvacSurrndgsTReForRowThrdAtFlrRi/1 -> HvacMdlAirTDistbnDynFildFrnt_Runnable_sys/70(HvacSurrndgsTReForRowThrdAtFlrRi_HvacSurrndgsTReForRowThrdAtFlrRi_read)
  HvacSurrndgsTReForRowThrdAtVentSideTopLe_HvacSurrndgsTReForRowThrdAtVentSideTopLe/1 -> HvacMdlAirTDistbnDynFildFrnt_Runnable_sys/71(HvacSurrndgsTReForRowThrdAtVentSideTopLe_HvacSurrndgsTReForRowThrdAtVentSideTopLe_read)
  HvacSurrndgsTReForRowThrdAtVentSideTopRi_HvacSurrndgsTReForRowThrdAtVentSideTopRi/1 -> HvacMdlAirTDistbnDynFildFrnt_Runnable_sys/72(HvacSurrndgsTReForRowThrdAtVentSideTopRi_HvacSurrndgsTReForRowThrdAtVentSideTopRi_read)
  HvacTReStatFildForRowSecAtVentSideTopLe_HvacTReStatFildForRowSecAtVentSideTopLe/1 -> HvacMdlAirTDistbnDynFildFrnt_Runnable_sys/73(HvacTReStatFildForRowSecAtVentSideTopLe_HvacTReStatFildForRowSecAtVentSideTopLe_read)
  HvacTReStatFildForRowSecAtVentSideTopRi_HvacTReStatFildForRowSecAtVentSideTopRi/1 -> HvacMdlAirTDistbnDynFildFrnt_Runnable_sys/74(HvacTReStatFildForRowSecAtVentSideTopRi_HvacTReStatFildForRowSecAtVentSideTopRi_read)
  HvacTReStatFildForRowThrdAtFlrLe_HvacTReStatFildForRowThrdAtFlrLe/1 -> HvacMdlAirTDistbnDynFildFrnt_Runnable_sys/75(HvacTReStatFildForRowThrdAtFlrLe_HvacTReStatFildForRowThrdAtFlrLe_read)
  HvacTReStatFildForRowThrdAtFlrRi_HvacTReStatFildForRowThrdAtFlrRi/1 -> HvacMdlAirTDistbnDynFildFrnt_Runnable_sys/76(HvacTReStatFildForRowThrdAtFlrRi_HvacTReStatFildForRowThrdAtFlrRi_read)
  HvacTReStatFildForRowThrdAtVentSideTopLe_HvacTReStatFildForRowThrdAtVentSideTopLe/1 -> HvacMdlAirTDistbnDynFildFrnt_Runnable_sys/77(HvacTReStatFildForRowThrdAtVentSideTopLe_HvacTReStatFildForRowThrdAtVentSideTopLe_read)
  HvacTReStatFildForRowThrdAtVentSideTopRi_HvacTReStatFildForRowThrdAtVentSideTopRi/1 -> HvacMdlAirTDistbnDynFildFrnt_Runnable_sys/78(HvacTReStatFildForRowThrdAtVentSideTopRi_HvacTReStatFildForRowThrdAtVentSideTopRi_read)
  HvacMdlAirTDistbnDynFildFrnt_Runnable_sys/1(HvacSnsrTReFildForRowSecAtVentSideTopLeRaw_HvacSnsrTReFildForRowSecAtVentSideTopLeRaw_write) -> HvacSnsrTReFildForRowSecAtVentSideTopLeRaw_HvacSnsrTReFildForRowSecAtVentSideTopLeRaw/1
  HvacMdlAirTDistbnDynFildFrnt_Runnable_sys/2(HvacSnsrTReFildForRowSecAtVentSideTopRiRaw_HvacSnsrTReFildForRowSecAtVentSideTopRiRaw_write) -> HvacSnsrTReFildForRowSecAtVentSideTopRiRaw_HvacSnsrTReFildForRowSecAtVentSideTopRiRaw/1
  HvacMdlAirTDistbnDynFildFrnt_Runnable_sys/3(HvacSnsrTReFildForRowThrdAtFlrLeRaw_HvacSnsrTReFildForRowThrdAtFlrLeRaw_write) -> HvacSnsrTReFildForRowThrdAtFlrLeRaw_HvacSnsrTReFildForRowThrdAtFlrLeRaw/1
  HvacMdlAirTDistbnDynFildFrnt_Runnable_sys/4(HvacSnsrTReFildForRowThrdAtFlrRiRaw_HvacSnsrTReFildForRowThrdAtFlrRiRaw_write) -> HvacSnsrTReFildForRowThrdAtFlrRiRaw_HvacSnsrTReFildForRowThrdAtFlrRiRaw/1
  HvacMdlAirTDistbnDynFildFrnt_Runnable_sys/5(HvacSnsrTReFildForRowThrdAtVentSideTopLeRaw_HvacSnsrTReFildForRowThrdAtVentSideTopLeRaw_write) -> HvacSnsrTReFildForRowThrdAtVentSideTopLeRaw_HvacSnsrTReFildForRowThrdAtVentSideTopLeRaw/1
  HvacMdlAirTDistbnDynFildFrnt_Runnable_sys/6(HvacSnsrTReFildForRowThrdAtVentSideTopRiRaw_HvacSnsrTReFildForRowThrdAtVentSideTopRiRaw_write) -> HvacSnsrTReFildForRowThrdAtVentSideTopRiRaw_HvacSnsrTReFildForRowThrdAtVentSideTopRiRaw/1
  HvacMdlAirTDistbnDynFildFrnt_Runnable_sys/7(HvacTReForRowSecAtVentSideTopLe_HvacTReForRowSecAtVentSideTopLe_write) -> HvacTReForRowSecAtVentSideTopLe_HvacTReForRowSecAtVentSideTopLe/1
  HvacMdlAirTDistbnDynFildFrnt_Runnable_sys/8(HvacTReForRowSecAtVentSideTopRi_HvacTReForRowSecAtVentSideTopRi_write) -> HvacTReForRowSecAtVentSideTopRi_HvacTReForRowSecAtVentSideTopRi/1
  HvacMdlAirTDistbnDynFildFrnt_Runnable_sys/9(HvacTReForRowThrdAtFlrLe_HvacTReForRowThrdAtFlrLe_write) -> HvacTReForRowThrdAtFlrLe_HvacTReForRowThrdAtFlrLe/1
  HvacMdlAirTDistbnDynFildFrnt_Runnable_sys/10(HvacTReForRowThrdAtFlrRi_HvacTReForRowThrdAtFlrRi_write) -> HvacTReForRowThrdAtFlrRi_HvacTReForRowThrdAtFlrRi/1
  HvacMdlAirTDistbnDynFildFrnt_Runnable_sys/11(HvacTReForRowThrdAtVentSideTopLe_HvacTReForRowThrdAtVentSideTopLe_write) -> HvacTReForRowThrdAtVentSideTopLe_HvacTReForRowThrdAtVentSideTopLe/1
  HvacMdlAirTDistbnDynFildFrnt_Runnable_sys/12(HvacTReForRowThrdAtVentSideTopRi_HvacTReForRowThrdAtVentSideTopRi_write) -> HvacTReForRowThrdAtVentSideTopRi_HvacTReForRowThrdAtVentSideTopRi/1
  HvacMdlAirTDistbnDynFildFrnt_Runnable_sys/13(HvacSnsrTFildForRowSecAtVentSideLe_HvacSnsrTFildForRowSecAtVentSideLe_write) -> HvacSnsrTFildForRowSecAtVentSideLe_HvacSnsrTFildForRowSecAtVentSideLe/1
  HvacMdlAirTDistbnDynFildFrnt_Runnable_sys/14(HvacSnsrTFildForRowSecAtVentSideRi_HvacSnsrTFildForRowSecAtVentSideRi_write) -> HvacSnsrTFildForRowSecAtVentSideRi_HvacSnsrTFildForRowSecAtVentSideRi/1
  HvacMdlAirTDistbnDynFildFrnt_Runnable_sys/15(HvacTFrntForRowFirstAtDefrstLe_HvacTFrntForRowFirstAtDefrstLe_write) -> HvacTFrntForRowFirstAtDefrstLe_HvacTFrntForRowFirstAtDefrstLe/1
  HvacMdlAirTDistbnDynFildFrnt_Runnable_sys/16(HvacTFrntForRowFirstAtDefrstRi_HvacTFrntForRowFirstAtDefrstRi_write) -> HvacTFrntForRowFirstAtDefrstRi_HvacTFrntForRowFirstAtDefrstRi/1
  HvacMdlAirTDistbnDynFildFrnt_Runnable_sys/17(HvacTFrntForRowFirstAtDefrstSideLe_HvacTFrntForRowFirstAtDefrstSideLe_write) -> HvacTFrntForRowFirstAtDefrstSideLe_HvacTFrntForRowFirstAtDefrstSideLe/1
  HvacMdlAirTDistbnDynFildFrnt_Runnable_sys/18(HvacTFrntForRowFirstAtDefrstSideRi_HvacTFrntForRowFirstAtDefrstSideRi_write) -> HvacTFrntForRowFirstAtDefrstSideRi_HvacTFrntForRowFirstAtDefrstSideRi/1
  HvacMdlAirTDistbnDynFildFrnt_Runnable_sys/19(HvacTFrntForRowFirstAtFlrLe_HvacTFrntForRowFirstAtFlrLe_write) -> HvacTFrntForRowFirstAtFlrLe_HvacTFrntForRowFirstAtFlrLe/1
  HvacMdlAirTDistbnDynFildFrnt_Runnable_sys/20(HvacTFrntForRowFirstAtFlrRi_HvacTFrntForRowFirstAtFlrRi_write) -> HvacTFrntForRowFirstAtFlrRi_HvacTFrntForRowFirstAtFlrRi/1
  HvacMdlAirTDistbnDynFildFrnt_Runnable_sys/21(HvacTFrntForRowFirstAtVentLe_HvacTFrntForRowFirstAtVentLe_write) -> HvacTFrntForRowFirstAtVentLe_HvacTFrntForRowFirstAtVentLe/1
  HvacMdlAirTDistbnDynFildFrnt_Runnable_sys/22(HvacTFrntForRowFirstAtVentRi_HvacTFrntForRowFirstAtVentRi_write) -> HvacTFrntForRowFirstAtVentRi_HvacTFrntForRowFirstAtVentRi/1
  HvacMdlAirTDistbnDynFildFrnt_Runnable_sys/23(HvacTFrntForRowFirstAtVentSideLe_HvacTFrntForRowFirstAtVentSideLe_write) -> HvacTFrntForRowFirstAtVentSideLe_HvacTFrntForRowFirstAtVentSideLe/1
  HvacMdlAirTDistbnDynFildFrnt_Runnable_sys/24(HvacTFrntForRowFirstAtVentSideRi_HvacTFrntForRowFirstAtVentSideRi_write) -> HvacTFrntForRowFirstAtVentSideRi_HvacTFrntForRowFirstAtVentSideRi/1
  HvacMdlAirTDistbnDynFildFrnt_Runnable_sys/25(HvacTFrntForRowSecAtFlrLe_HvacTFrntForRowSecAtFlrLe_write) -> HvacTFrntForRowSecAtFlrLe_HvacTFrntForRowSecAtFlrLe/1
  HvacMdlAirTDistbnDynFildFrnt_Runnable_sys/26(HvacTFrntForRowSecAtFlrRi_HvacTFrntForRowSecAtFlrRi_write) -> HvacTFrntForRowSecAtFlrRi_HvacTFrntForRowSecAtFlrRi/1
  HvacMdlAirTDistbnDynFildFrnt_Runnable_sys/27(HvacTFrntForRowSecAtVentLe_HvacTFrntForRowSecAtVentLe_write) -> HvacTFrntForRowSecAtVentLe_HvacTFrntForRowSecAtVentLe/1
  HvacMdlAirTDistbnDynFildFrnt_Runnable_sys/28(HvacTFrntForRowSecAtVentRi_HvacTFrntForRowSecAtVentRi_write) -> HvacTFrntForRowSecAtVentRi_HvacTFrntForRowSecAtVentRi/1
  HvacMdlAirTDistbnDynFildFrnt_Runnable_sys/29(HvacTFrntForRowSecAtVentSideLe_HvacTFrntForRowSecAtVentSideLe_write) -> HvacTFrntForRowSecAtVentSideLe_HvacTFrntForRowSecAtVentSideLe/1
  HvacMdlAirTDistbnDynFildFrnt_Runnable_sys/30(HvacTFrntForRowSecAtVentSideRi_HvacTFrntForRowSecAtVentSideRi_write) -> HvacTFrntForRowSecAtVentSideRi_HvacTFrntForRowSecAtVentSideRi/1
  HvacMdlAirTDistbnDynFildFrnt_Runnable_sys/31(IntrpnFacForTChForRowFirstLe_IntrpnFacForTChForRowFirstLe_write) -> IntrpnFacForTChForRowFirstLe_IntrpnFacForTChForRowFirstLe/1
  HvacMdlAirTDistbnDynFildFrnt_Runnable_sys/32(IntrpnFacForTChForRowFirstRi_IntrpnFacForTChForRowFirstRi_write) -> IntrpnFacForTChForRowFirstRi_IntrpnFacForTChForRowFirstRi/1
  HvacMdlAirTDistbnDynFildFrnt_Runnable_sys/33(IntrpnFacForTChForRowSecLe_IntrpnFacForTChForRowSecLe_write) -> IntrpnFacForTChForRowSecLe_IntrpnFacForTChForRowSecLe/1
  HvacMdlAirTDistbnDynFildFrnt_Runnable_sys/34(IntrpnFacForTChForRowSecRi_IntrpnFacForTChForRowSecRi_write) -> IntrpnFacForTChForRowSecRi_IntrpnFacForTChForRowSecRi/1
  HvacMdlAirTDistbnDynFildFrnt_Runnable/1 -> HvacMdlAirTDistbnDynFildFrnt_Runnable_sys/Trigger

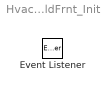
== system HvacMdlAirTDistbnDynFildFrnt/HvacMdlAirTDistbnDynFildFrnt_Init ==
  Event Listener : EventListener  EventName=reset
